simulink model "manas_spec_244b"  (R2022a)
settings: Solver=VariableStepDiscrete  StartTime=0.0  StopTime=524288  RelTol=1e-3
undefined symbols (not defined in the file): pfb_coeff_gen_calc
libraries: hdlBasic x10110, casper_library_misc x41, casper_library_delays x30, xps_library x3, xbsReplacements_r4 x2, xbsIndex_r4 x1, simulink x1
(part 89 of 128)

== system manas_spec_244b/fft_wideband_real/fft_direct/butterfly0_0/twiddle/coeff_gen/cosin ==
  theta : Inport
  misci : Inport  Port=2
  Constant4 : hdlBasic/Constant  const=0  gui_display_data_type=Fixed-point  arith_type=Unsigned  n_bits=1  bin_pt=0  preci_type=Single  exp_width=8  frac_width=24  explicit_period=on  period=1  XmcBlockStatus=1  maskContainerNames=tabcontainer1  block_type=constant  block_config=sysgen:Constant_config
  Constant5 : hdlBasic/Constant  const=0  gui_display_data_type=Fixed-point  arith_type=Unsigned  n_bits=1  bin_pt=0  preci_type=Single  exp_width=8  frac_width=24  explicit_period=on  period=1  XmcBlockStatus=1  maskContainerNames=tabcontainer1  block_type=constant  block_config=sysgen:Constant_config
  Delay : hdlBasic/Delay  rst=off  en=off  latency=bram_latency  reg_retiming=on  XmcBlockStatus=1  maskContainerNames=group1  block_type=delay  block_config=sysgen:Delay_config
  Delay10 : hdlBasic/Delay  rst=off  en=off  latency=bram_latency  reg_retiming=on  XmcBlockStatus=1  maskContainerNames=group1  block_type=delay  block_config=sysgen:Delay_config
  Delay8 : hdlBasic/Delay  rst=off  en=off  latency=bram_latency  reg_retiming=on  XmcBlockStatus=1  maskContainerNames=group1  block_type=delay  block_config=sysgen:Delay_config
  ROM : hdlBasic/ROM  depth=2^(14)  initVector=[34359476224 34359738343 34359738318 34359738293 34359738267 34359738242 34359738217 34359738192 34359738167 34359738142 34359738117 34359738092 34359738066 34359738041 34359738016 34359737991 34359737966 34359737941 34359737916 34359737890 34359737865 34359737840 34359737815 34359737790 34359737765 34359475596 34359475571 34359475545 34359475520 34359475495 34359475470 34359475445 34359475420 34359213251 34359213225 34359213200 34359213175 34359213150 34359213125 34358950956 34358950931 34358950906 34358950880 34358950855 34358688686 34358688661 34358688636 34358688611 34358426442 34358426417 34358426391 34358426366 34358164197 34358164172 34358164147 34358164122 34357901953 34357901927 34357901902 34357901877 34357639708 34357639683 34357639658 34357377489 34357377464 34357377438 34357377413 34357115244 34357115219 34357115194 34356853025 34356853000 34356852975 34356590805 34356590780 34356328611 34356328586 34356328561 34356066392 34356066367 34356066341 34355804172 34355804147 34355541978 34355541953 34355541928 34355279759 34355279734 34355017564 34355017539 34354755370 34354755345 34354755320 34354493151 34354493126 34354230957 34354230931 34353968762 34353968737 34353706568 34353706543 34353444374 34353444349 34353182179 34353182154 34352919985 34352919960 34352657791 34352657766 34352395597 34352395572 34352133402 34352133377 34351871208 34351871183 34351609014 34351608989 34351346820 34351084651 34351084625 34350822456 34350822431 34350560262 34350560237 34350298068 34350035899 34350035874 34349773704 34349773679 34349511510 34349249341 34349249316 34348987147 34348724978 34348724953 34348462783 34348200614 34348200589 34347938420 34347676251 34347676226 34347414057 34347151888 34347151862 34346889693 34346627524 34346627499 34346365330 34346103161 34346103136 34345840967 34345578797 34345316628 34345316603 34345054434 34344792265 34344530096 34344530071 34344267902 34344005733 34343743563 34343743538 34343481369 34343219200 34342957031 34342694862 34342694837 34342432668 34342170498 34341908329 34341646160 34341646135 34341383966 34341121797 34340859628 34340597459 34340335289 34340335264 34340073095 34339810926 34339548757 34339286588 34339024419 34338762250 34338500081 34338500055 34338237886 34337975717 34337713548 34337451379 34337189210 34336927041 34336664872 34336402702 34336140533 34335878364 34335616195 34335354026 34335354001 34335091832 34334829663 34334567494 34334305324 34334043155 34333780986 34333518817 34333256648 34332994479 34332732310 34332470141 34332207972 34331945802 34331683633 34331421464 34331159295 34330897126 34330634957 34330372788 34330110619 34329586306 34329324136 34329061967 34328799798 34328537629 34328275460 34328013291 34327751122 34327488953 34327226784 34326964614 34326702445 34326178132 34325915963 34325653794 34325391625 34325129456 34324867287 34324605118 34324342948 34323818635 34323556466 34323294297 34323032128 34322769959 34322507790 34321983477 34321721308 34321459139 34321196969 34320934800 34320410487 34320148318 34319886149 34319623980 34319361811 34318837498 34318575329 34318313159 34318050990 34317526677 34317264508 34317002339 34316740170 34316215857 34315953688 34315691519 34315429350 34314905036 34314642867 34314380698 34313856385 34313594216 34313332047 34312807734 34312545565 34312283396 34311759083 34311496914 34311234744 34310710431 34310448262 34310186093 34309661780 34309399611 34309137442 34308613129 34308350960 34308088791 34307564477 34307302308 34306777995 34306515826 34306253657 34305729344 34305467175 34304942862 34304680693 34304418524 34303894211 34303632041 34303107728 34302845559 34302321246 34302059077 34301534764 34301272595 34300748282 34300486113 34299961800 34299699631 34299437461 34298913148 34298650979 34298126666 34297602353 34297340184 34296815871 34296553702 34296029389 34295767220 34295242907 34294980737 34294456424 34294194255 34293669942 34293407773 34292883460 34292359147 34292096978 34291572665 34291310496 34290786183 34290261870 ...(+190900 chars)]  distributed_mem=Block RAM  rst=off  init_reg=0  en=off  latency=bram_latency  gui_display_data_type=Fixed-point  arith_type=Unsigned  n_bits=n_bits*2  bin_pt=0  preci_type=Single  exp_width=8  frac_width=24  optimize=Speed  use_rpm=on  simulation_type=fast_sim  XmcBlockStatus=1  maskContainerNames=tabcontainer1  block_type=sprom  block_config=sysgen:SPROM_config
  Terminator : Terminator
  Terminator1 : Terminator
  Terminator4 : Terminator
  add_convert0 : Subsystem
  add_convert1 : Subsystem
  assert : hdlBasic/Assert  assert_type=on  type_source=Explicitly  gui_display_data_type=Fixed-point  arith_type=Unsigned  n_bits=14  bin_pt=0  preci_type=Single  exp_width=8  frac_width=24  assert_rate=off  rate_source=Explicitly  period=1  output_port=on  XmcBlockStatus=0  maskContainerNames=group1  block_type=assert  block_config=sysgen:assert_config  block_width=609  block_height=900
  c_to_ri : casper_library_misc/c_to_ri  n_bits=n_bits  bin_pt=bin_pt
      settings: SourceProductName=CASPER DSP Blockset
  invert0 : Subsystem  mask{n_bits=n_bits, bin_pt=bin_pt, neg_latency=neg_latency, mux_latency=mux_latency}
      mask prompts: n_bits "bit width"; bin_pt "binary point"; neg_latency "negate latency"; mux_latency "mux latency"
  invert1 : Subsystem  mask{n_bits=n_bits, bin_pt=bin_pt, neg_latency=neg_latency, mux_latency=mux_latency}
      mask prompts: n_bits "bit width"; bin_pt "binary point"; neg_latency "negate latency"; mux_latency "mux latency"
  cos : Outport
  -sin : Outport  Port=2
  misco : Outport  Port=3
  wires:
  theta/1 -> assert/1
